simulink model "SdbRxSigProc"  (R2022b)
settings: Solver=FixedStepDiscrete  StartTime=0.0  StopTime=10.0  FixedStep=0.05  RelTol=1e-3
(part 1 of 4)
settings Model: Creator=blue.ge  LastModifiedBy=blue.ge
settings DataIOCC: SaveFormat=StructureWithTime
settings OptimizationCC: DefaultParameterBehavior=Inlined  EnhancedBackFolding=on  PassReuseOutputArgsAs=Individual arguments  ZeroExternalMemoryAtStartup=off  ZeroInternalMemoryAtStartup=off  OptimizeBlockOrder=speed  DifferentSizesBufferReuse=on  OptimizationCustomize=off  EfficientTunableParamExpr=on
settings DebuggingCC: ParameterTunabilityLossMsg=error
settings HardwareCC: ProdLongLongMode=on
settings ModelReferenceCC: ModelReferenceNumInstancesAllowed=Single
settings SFSimCC: MATLABDynamicMemAlloc=off
settings RTWCC: Description=AUTOSAR  SystemTargetFile=autosar.tlc  GenCodeOnly=on  TemplateMakefile=ert_default_tmf
settings CodeAppCC: ForceParamTrailComments=on  IgnoreCustomStorageClasses=off  OperatorAnnotations=on  SimulinkDataObjDesc=on  SFDataObjDesc=on  InsertBlockDesc=on
settings STFCustomTargetCC: TargetLangStandard=C99 (ISO)  MultiwordLength=256  ParMdlRefBuildCompliant=on  CompOptLevelCompliant=on  IncludeMdlTerminateFcn=off  CombineOutputUpdateFcns=on  SuppressErrorStatus=on  ERTCustomFileBanners=on  SupportNonFinite=off  SupportComplex=off  SupportContinuousTime=off  SupportNonInlinedSFcns=off  RemoveResetFunc=on  MATLABClassNameForMDSCustomization=Simulink.SoftwareTarget.ERTCustomization  AutosarCompliant=on  UseToolchainInfoCompliant=on  LookupTableObjectStructAxisOrder=2,1,3,4,...  LUTObjectStructOrderEvenSpacing=Size,Table,Breakpoints  SystemTargetFile=autosar.tlc
settings ERTTargetCC: TargetLangStandard=C99 (ISO)  ConcurrentExecutionCompliant=off  IncludeMdlTerminateFcn=off  SuppressErrorStatus=on  SupportNonFinite=off  SupportComplex=off  ModelStepFunctionPrototypeControlCompliant=off  CPPClassGenCompliant=off  AutosarCompliant=on  LookupTableObjectStructAxisOrder=2,1,3,4,...  LUTObjectStructOrderEvenSpacing=Size,Table,Breakpoints  InlinedParameterPlacement=NonHierarchical  ERTCustomFileTemplate=example_file_process.tlc

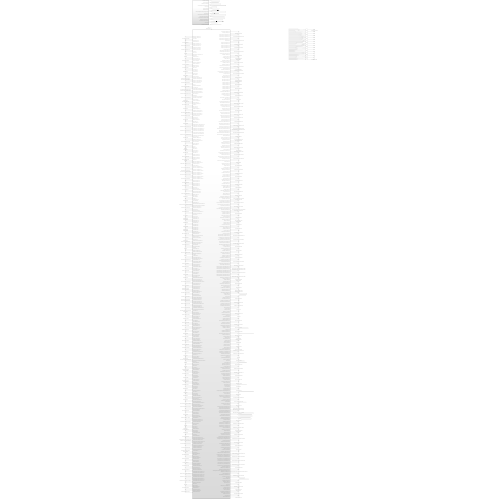
[thumbnail: Simulink's stored preview of the top-level diagram]
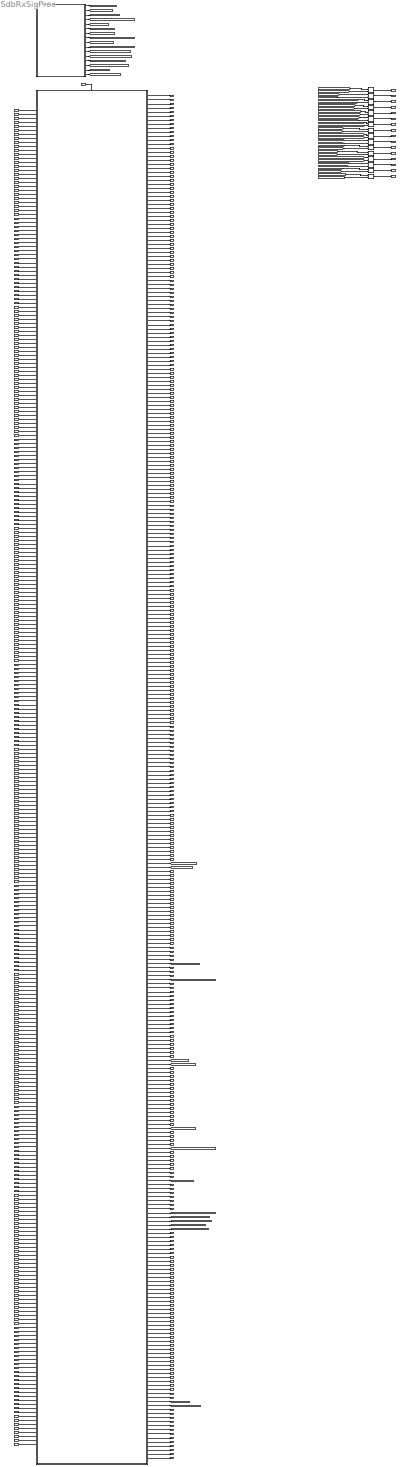
== system SdbRxSigProc ==
  SdbRxSigProc_Runnable : Inport  SampleTime=0.05  OutputFunctionCall=on
  ActvnOfWshrFrntSafe_ActvnOfWshrFrntSafe : Inport  Port=2  OutDataTypeStr=Enum: OnOffCrit1  PortDimensions=1
  AirTFromHeatgEstimd_AirTFromHeatgEstimd : Inport  Port=3  OutMin=-256  OutMax=255.9  OutDataTypeStr=T2  PortDimensions=1
  AmbTRaw_AmbTRaw : Inport  Port=4  OutDataTypeStr=Bus: AmbTWithQly  PortDimensions=1  BusOutputAsStruct=on
  BltLockStAtDrvr_BltLockStAtDrvr : Inport  Port=5  OutDataTypeStr=Bus: BltLockStFrnt  PortDimensions=1  BusOutputAsStruct=on
  BltLockStAtPass_BltLockStAtPass : Inport  Port=6  OutDataTypeStr=Bus: BltLockStFrnt  PortDimensions=1  BusOutputAsStruct=on
  BltLockStAtRowSecLe_BltLockStAtRowSecLe : Inport  Port=7  OutDataTypeStr=Bus: BltLockStRe  PortDimensions=1  BusOutputAsStruct=on
  BltLockStAtRowSecMid_BltLockStAtRowSecMid : Inport  Port=8  OutDataTypeStr=Bus: BltLockStRe  PortDimensions=1  BusOutputAsStruct=on
  BltLockStAtRowSecRi_BltLockStAtRowSecRi : Inport  Port=9  OutDataTypeStr=Bus: BltLockStRe  PortDimensions=1  BusOutputAsStruct=on
  BltLockStAtRowThrdLe_BltLockStAtRowThrdLe : Inport  Port=10  OutDataTypeStr=Bus: BltLockStRe  PortDimensions=1  BusOutputAsStruct=on
  BltLockStAtRowThrdRi_BltLockStAtRowThrdRi : Inport  Port=11  OutDataTypeStr=Bus: BltLockStRe  PortDimensions=1  BusOutputAsStruct=on
  BookTrvlClimaActvReq_BookTrvlClimaActvReq : Inport  Port=12  OutDataTypeStr=Enum: OnOffNoReq  PortDimensions=1
  CamSts_CamSts : Inport  Port=13  OutDataTypeStr=Bus: CamStsGrp  PortDimensions=1  BusOutputAsStruct=on
  CarTiGlb_CarTiGlb : Inport  Port=14  OutDataTypeStr=CarTiGlb  PortDimensions=1
  ClimaCfgForSnsrHumPrsnt1_ClimaCfgForSnsrHumPrsnt1 : Inport  Port=15  OutDataTypeStr=Boolean  PortDimensions=1
  ClimaCmd_ClimaCmd : Inport  Port=16  OutDataTypeStr=Enum: OkNotOk  PortDimensions=1
  ClimaPwrAllwd_ClimaPwrAllwd : Inport  Port=17  OutMin=0  OutMax=2047  OutDataTypeStr=Pwr30  PortDimensions=1
  CmpmtInAirPmAQI_CmpmtInAirPmAQI : Inport  Port=18  OutDataTypeStr=Enum: CmpmtAirPmLvl  PortDimensions=1
  CmpmtInAirPmDnsty_CmpmtInAirPmDnsty : Inport  Port=19  OutDataTypeStr=UInt10  PortDimensions=1
  CmptmtCoolgSts_CmptmtCoolgSts : Inport  Port=20  OutDataTypeStr=Enum: CmptmtCoolgSts  PortDimensions=1
  CmptmtFrntWindDewT_CmptmtFrntWindDewT : Inport  Port=21  OutDataTypeStr=TByCmptmtSnsrRelHum  PortDimensions=1
  CmptmtFrntWindT_CmptmtFrntWindT : Inport  Port=22  OutDataTypeStr=TByCmptmtSnsrRelHum  PortDimensions=1
  CmptmtRelHum_CmptmtRelHum : Inport  Port=23  OutMin=0  OutMax=100  OutDataTypeStr=Perc2  PortDimensions=1
  CoolgLoadGroup_CoolgLoadGroup : Inport  Port=24  OutDataTypeStr=Bus: CoolgLoad1  PortDimensions=1  BusOutputAsStruct=on
  DefrstSts_DefrstSts : Inport  Port=25  OutDataTypeStr=Bus: DefrstSts  PortDimensions=1  BusOutputAsStruct=on
  DoorDrvrSts_DoorDrvrSts : Inport  Port=26  OutDataTypeStr=Enum: DoorSts2  PortDimensions=1
  DoorLeReSts_DoorLeReSts : Inport  Port=27  OutDataTypeStr=Enum: DoorSts2  PortDimensions=1
  DoorPassSts_DoorPassSts : Inport  Port=28  OutDataTypeStr=Enum: DoorSts2  PortDimensions=1
  DoorRiReSts_DoorRiReSts : Inport  Port=29  OutDataTypeStr=Enum: DoorSts2  PortDimensions=1
  DrvModReq_DrvModReq : Inport  Port=30  OutDataTypeStr=Enum: DrvModReqType1  PortDimensions=1
  DrvrAirCdnrSetg_DrvrAirCdnrSetg : Inport  Port=31  OutDataTypeStr=Bus: DrvrAirCdnrSetg  PortDimensions=1  BusOutputAsStruct=on
  DrvrSeatHeatgActlPwrCns_DrvrSeatHeatgActlPwrCns : Inport  Port=32  OutDataTypeStr=UInt8  PortDimensions=1
  DrvrSeatHeatgDesPwrCns_DrvrSeatHeatgDesPwrCns : Inport  Port=33  OutDataTypeStr=UInt8  PortDimensions=1
  DrvrSeatVentnActlPwrCns_DrvrSeatVentnActlPwrCns : Inport  Port=34  OutDataTypeStr=UInt8  PortDimensions=1
  DrvrSeatVentnDesPwrCns_DrvrSeatVentnDesPwrCns : Inport  Port=35  OutDataTypeStr=UInt8  PortDimensions=1
  EgyCnsAllwdForClima_EgyCnsAllwdForClima : Inport  Port=36  OutDataTypeStr=Egy2  PortDimensions=1
  EngT_EngT : Inport  Port=37  OutDataTypeStr=Bus: EngT  PortDimensions=1  BusOutputAsStruct=on
  HMIClimaEgySaveReq_HMIClimaEgySaveReq : Inport  Port=38  OutDataTypeStr=Enum: VentnActr01BlckInd  PortDimensions=1
  HpcDeiceReq_HpcDeiceReq : Inport  Port=39  OutDataTypeStr=Enum: DeiceReq  PortDimensions=1
  HvacFanSts2HvacFanBattI2_HvacFanSts2HvacFanBattI2 : Inport  Port=40  OutMin=0  OutMax=63.25  OutDataTypeStr=BlowerReI  PortDimensions=1
  HvacFanSts2HvacFanMotU2_HvacFanSts2HvacFanMotU2 : Inport  Port=41  OutMin=0  OutMax=25.400000000000002  OutDataTypeStr=U8  PortDimensions=1
  HvacFanSts3HvacFanBattI_HvacFanSts3HvacFanBattI : Inport  Port=42  OutDataTypeStr=BlowerBattI  PortDimensions=1
  HvacFanSts3HvacFanSpdFd_HvacFanSts3HvacFanSpdFd : Inport  Port=43  OutDataTypeStr=BlowerSpd  PortDimensions=1
  HvPwrEgyPrio_HvPwrEgyPrio : Inport  Port=44  OutDataTypeStr=Enum: HvPwrEgyPrio  PortDimensions=1
  IHUAPSts_IHUAPSts : Inport  Port=45  OutDataTypeStr=Enum: OnOff1  PortDimensions=1
  IncomingAirQlyCtrlFromHmi_IncomingAirQlyCtrlFromHmi : Inport  Port=46  OutDataTypeStr=Enum: OnOff1  PortDimensions=1
  IncomingAirQlyPopUpReq_IncomingAirQlyPopUpReq : Inport  Port=47  OutDataTypeStr=Enum: OnOff1  PortDimensions=1
  InPm25FanErr_InPm25FanErr : Inport  Port=48  OutDataTypeStr=Enum: FanErr  PortDimensions=1
  InPm25SnsrElecErr_InPm25SnsrElecErr : Inport  Port=49  OutDataTypeStr=Enum: PmSnsrErrSts  PortDimensions=1
  InPm25SnsrSts_InPm25SnsrSts : Inport  Port=50  OutDataTypeStr=Enum: PmSnsrSts  PortDimensions=1
  InteCleanUnpleSmell_InteCleanUnpleSmell : Inport  Port=51  OutDataTypeStr=Enum: OnOff1  PortDimensions=1
  IsgMotT_IsgMotT : Inport  Port=52  OutDataTypeStr=T3  PortDimensions=1
  LeSolarData_LeSolarData : Inport  Port=53  OutDataTypeStr=Sunload2D  PortDimensions=1
  LockgCenSts_LockgCenSts : Inport  Port=54  OutDataTypeStr=Bus: LockgCenSts3  PortDimensions=1  BusOutputAsStruct=on
  OnBdChrgrHndlSts1_OnBdChrgrHndlSts1 : Inport  Port=55  OutDataTypeStr=Enum: OnBdChrgrHndlSts1  PortDimensions=1
  PassSeatHeatgActlPwrCns_PassSeatHeatgActlPwrCns : Inport  Port=56  OutDataTypeStr=UInt8  PortDimensions=1
  PassSeatHeatgDesPwrCns_PassSeatHeatgDesPwrCns : Inport  Port=57  OutDataTypeStr=UInt8  PortDimensions=1
  PassSeatVentnActlPwrCns_PassSeatVentnActlPwrCns : Inport  Port=58  OutDataTypeStr=UInt8  PortDimensions=1
  PassSeatVentnDesPwrCns_PassSeatVentnDesPwrCns : Inport  Port=59  OutDataTypeStr=UInt8  PortDimensions=1
  PostDrvgClimaReq_PostDrvgClimaReq : Inport  Port=60  OutDataTypeStr=Enum: OnOff1  PortDimensions=1
  RainfallAmnt_RainfallAmnt : Inport  Port=61  OutDataTypeStr=Enum: AmntSnsr  PortDimensions=1
  RelHumSnsrQf_RelHumSnsrQf : Inport  Port=62  OutDataTypeStr=Enum: DataQf  PortDimensions=1
  RemStrtHvCtrlReq_RemStrtHvCtrlReq : Inport  Port=63  OutDataTypeStr=Bus: ErsCmdRec  PortDimensions=1  BusOutputAsStruct=on
  RiSolarData_RiSolarData : Inport  Port=64  OutDataTypeStr=Sunload2D  PortDimensions=1
  SceneModSeld_SceneModSeld : Inport  Port=65  OutDataTypeStr=Enum: PatSeld  PortDimensions=1
  SeatHeatDurgClimaEnadFromTelm_SeatHeatDurgClimaEnadFromTelm : Inport  Port=66  OutDataTypeStr=Enum: SeatHeatDurgClimaEnad  PortDimensions=1
  SecRowSeatHeatgActlPwrCnsLe_SecRowSeatHeatgActlPwrCnsLe : Inport  Port=67  OutDataTypeStr=UInt8  PortDimensions=1
  SecRowSeatHeatgActlPwrCnsRi_SecRowSeatHeatgActlPwrCnsRi : Inport  Port=68  OutDataTypeStr=UInt8  PortDimensions=1
  SecRowSeatHeatgDesPwrCnsLe_SecRowSeatHeatgDesPwrCnsLe : Inport  Port=69  OutDataTypeStr=UInt8  PortDimensions=1
  SecRowSeatHeatgDesPwrCnsRi_SecRowSeatHeatgDesPwrCnsRi : Inport  Port=70  OutDataTypeStr=UInt8  PortDimensions=1
  SecRowSeatVentnActlPwrCnsLe_SecRowSeatVentnActlPwrCnsLe : Inport  Port=71  OutDataTypeStr=UInt8  PortDimensions=1
  SecRowSeatVentnActlPwrCnsRi_SecRowSeatVentnActlPwrCnsRi : Inport  Port=72  OutDataTypeStr=UInt8  PortDimensions=1
  SecRowSeatVentnDesPwrCnsLe_SecRowSeatVentnDesPwrCnsLe : Inport  Port=73  OutDataTypeStr=UInt8  PortDimensions=1
  SecRowSeatVentnDesPwrCnsRi_SecRowSeatVentnDesPwrCnsRi : Inport  Port=74  OutDataTypeStr=UInt8  PortDimensions=1
  SolarSnsrVluQf_SolarSnsrVluQf : Inport  Port=75  OutDataTypeStr=Enum: DataQf  PortDimensions=1
  SteerWhlHeatgDesPwr_SteerWhlHeatgDesPwr : Inport  Port=76  OutDataTypeStr=UInt8  PortDimensions=1
  SteerWhlHeatgPwrAct_SteerWhlHeatgPwrAct : Inport  Port=77  OutDataTypeStr=UInt8  PortDimensions=1
  StrtRemSts1WdStrtRemSts_StrtRemSts1WdStrtRemSts : Inport  Port=78  OutDataTypeStr=Enum: StrtRemSts1  PortDimensions=1
  SunCurtPosnSts_SunCurtPosnSts : Inport  Port=79  OutDataTypeStr=Enum: WinAndRoofAndCurtPosnTyp  PortDimensions=1
  SunRoofPosnSts_SunRoofPosnSts : Inport  Port=80  OutDataTypeStr=Enum: WinAndRoofAndCurtPosnTyp  PortDimensions=1
  TelmPM25Req_TelmPM25Req : Inport  Port=81  OutDataTypeStr=Enum: ReqSts1  PortDimensions=1
  TrSts_TrSts : Inport  Port=82  OutDataTypeStr=Enum: DoorSts2  PortDimensions=1
  VehBattU_VehBattU : Inport  Port=83  OutDataTypeStr=Bus: SysU  PortDimensions=1  BusOutputAsStruct=on
  VehSpdLgt_VehSpdLgt : Inport  Port=84  OutDataTypeStr=Bus: VehSpdLgtSafe1  PortDimensions=1  BusOutputAsStruct=on
  VehTiAndData_VehTiAndData : Inport  Port=85  OutDataTypeStr=Bus: DateTi30  PortDimensions=1  BusOutputAsStruct=on
  WadeProtnSts_WadeProtnSts : Inport  Port=86  OutDataTypeStr=Enum: OnOff1  PortDimensions=1
  WinGlbCmd_WinGlbCmd : Inport  Port=87  OutDataTypeStr=Enum: OpenClsGlbCmd  PortDimensions=1
  WinPosnStsAtDrvr_WinPosnStsAtDrvr : Inport  Port=88  OutDataTypeStr=Enum: WinAndRoofAndCurtPosnTyp  PortDimensions=1
  WinPosnStsAtPass_WinPosnStsAtPass : Inport  Port=89  OutDataTypeStr=Enum: WinAndRoofAndCurtPosnTyp  PortDimensions=1
  WinPosnStsAtReLe_WinPosnStsAtReLe : Inport  Port=90  OutDataTypeStr=Enum: WinAndRoofAndCurtPosnTyp  PortDimensions=1
  WinPosnStsAtReRi_WinPosnStsAtReRi : Inport  Port=91  OutDataTypeStr=Enum: WinAndRoofAndCurtPosnTyp  PortDimensions=1
  WipgInfo_WipgInfo : Inport  Port=92  OutDataTypeStr=Bus: WipgInfo  PortDimensions=1  BusOutputAsStruct=on
  VehModMngtGlbSafe1_VehModMngtGlbSafe1 : Inport  Port=93  OutDataTypeStr=Bus: VehModMngtGlbSafe1  PortDimensions=1  BusOutputAsStruct=on
  VehModMngtGlbSafe1_VehModMngtGlbSafe1_IsUpdated : Inport  Port=94  OutDataTypeStr=boolean  PortDimensions=1
  AACAirClngErrSts_AACAirClngErrSts : Inport  Port=95  OutDataTypeStr=Enum: AACAirClngErrSts  PortDimensions=1
  AACAirClngSts_AACAirClngSts : Inport  Port=96  OutDataTypeStr=Enum: AACAirClngSts  PortDimensions=1
  AFUFragraCh1AvlTiPrec_AFUFragraCh1AvlTiPrec : Inport  Port=97  OutMin=0  OutMax=100  OutDataTypeStr=Perc9  PortDimensions=1
  AFUFragraCh1AvlTiPrec_AFUFragraCh1AvlTiPrec_IsUpdated : Inport  Port=98  OutDataTypeStr=boolean  PortDimensions=1
  AFUFragraCh2AvlTiPrec_AFUFragraCh2AvlTiPrec : Inport  Port=99  OutMin=0  OutMax=100  OutDataTypeStr=Perc9  PortDimensions=1
  AFUFragraCh2AvlTiPrec_AFUFragraCh2AvlTiPrec_IsUpdated : Inport  Port=100  OutDataTypeStr=boolean  PortDimensions=1
  AFUFragraCh3AvlTiPrec_AFUFragraCh3AvlTiPrec : Inport  Port=101  OutMin=0  OutMax=100  OutDataTypeStr=Perc9  PortDimensions=1
  AFUFragraCh3AvlTiPrec_AFUFragraCh3AvlTiPrec_IsUpdated : Inport  Port=102  OutDataTypeStr=boolean  PortDimensions=1
  AFUFragraCh4AvlTiPrec_AFUFragraCh4AvlTiPrec : Inport  Port=103  OutMin=0  OutMax=100  OutDataTypeStr=Perc9  PortDimensions=1
  AFUFragraCh4AvlTiPrec_AFUFragraCh4AvlTiPrec_IsUpdated : Inport  Port=104  OutDataTypeStr=boolean  PortDimensions=1
  AFUFragraCh5AvlTiPrec_AFUFragraCh5AvlTiPrec : Inport  Port=105  OutMin=0  OutMax=100  OutDataTypeStr=Perc9  PortDimensions=1
  AFUFragraCh5AvlTiPrec_AFUFragraCh5AvlTiPrec_IsUpdated : Inport  Port=106  OutDataTypeStr=boolean  PortDimensions=1
  AirFragCh1OvrdDy_AirFragCh1OvrdDy : Inport  Port=107  OutDataTypeStr=Bus: DateTi30  PortDimensions=1  BusOutputAsStruct=on
  AirFragCh2OvrdDy_AirFragCh2OvrdDy : Inport  Port=108  OutDataTypeStr=Bus: DateTi30  PortDimensions=1  BusOutputAsStruct=on
  AirFragCh3OvrdDy_AirFragCh3OvrdDy : Inport  Port=109  OutDataTypeStr=Bus: DateTi30  PortDimensions=1  BusOutputAsStruct=on
  AirFragCh4OvrdDy_AirFragCh4OvrdDy : Inport  Port=110  OutDataTypeStr=Bus: DateTi30  PortDimensions=1  BusOutputAsStruct=on
  AirFragCh5OvrdDy_AirFragCh5OvrdDy : Inport  Port=111  OutDataTypeStr=Bus: DateTi30  PortDimensions=1  BusOutputAsStruct=on
  AirPtcEnad_AirPtcEnad : Inport  Port=112  OutDataTypeStr=Boolean  PortDimensions=1
  AirPtcHVPwrActl_AirPtcHVPwrActl : Inport  Port=113  OutDataTypeStr=Pwr5  PortDimensions=1
  AirPtcHVPwrActlFristLe_AirPtcHVPwrActlFristLe : Inport  Port=114  OutMin=0  OutMax=20460  OutDataTypeStr=Pwr3  PortDimensions=1
  AirPtcHVPwrActlFristRi_AirPtcHVPwrActlFristRi : Inport  Port=115  OutMin=0  OutMax=20460  OutDataTypeStr=Pwr3  PortDimensions=1
  AirPtcHVPwrActlSecLe_AirPtcHVPwrActlSecLe : Inport  Port=116  OutMin=0  OutMax=20460  OutDataTypeStr=Pwr3  PortDimensions=1
  AirPtcModeReq_AirPtcModeReq : Inport  Port=117  OutDataTypeStr=Enum: AirPtcModeReq  PortDimensions=1
  AirSrcStsFb_AirSrcStsFb : Inport  Port=118  OutMin=0  OutMax=3  OutDataTypeStr=UInt2  PortDimensions=1
  AltitudeInfrom_AltitudeInfrom : Inport  Port=119  OutDataTypeStr=UInt15  PortDimensions=1
  AMBSts_AMBSts : Inport  Port=120  OutDataTypeStr=Bus: AMBStsgrp  PortDimensions=1  BusOutputAsStruct=on
  BackflushVlvErr_BackflushVlvErr : Inport  Port=121  OutMin=0  OutMax=3  OutDataTypeStr=UInt2  PortDimensions=1
  BusCurrentFb_BusCurrentFb : Inport  Port=122  OutDataTypeStr=PackCurrent  PortDimensions=1
  ChgFilElmWarn_ChgFilElmWarn : Inport  Port=123  OutMin=0  OutMax=3  OutDataTypeStr=UInt2  PortDimensions=1
  ClimaCfgForDrvrSeatVentWthSmf2_ClimaCfgForDrvrSeatVentWthSmf2 : Inport  Port=124  OutDataTypeStr=UInt8  PortDimensions=1
  ClimaCfgForPassSeatVentWthSmf2_ClimaCfgForPassSeatVentWthSmf2 : Inport  Port=125  OutDataTypeStr=UInt8  PortDimensions=1
  CmptmtOxygenConcQF_CmptmtOxygenConcQF : Inport  Port=126  OutDataTypeStr=Enum: Qf1  PortDimensions=1
  CmptmtOxygenConcVlu_CmptmtOxygenConcVlu : Inport  Port=127  OutMin=0  OutMax=100  OutDataTypeStr=Perc9  PortDimensions=1
  CoolgFanErr_CoolgFanErr : Inport  Port=128  OutMin=0  OutMax=3  OutDataTypeStr=UInt2  PortDimensions=1
  CourseDirFromNav_CourseDirFromNav : Inport  Port=129  OutDataTypeStr=Bus: NavDir  PortDimensions=1  BusOutputAsStruct=on
  CurrentCongestionInformation_CurrentCongestionInformation : Inport  Port=130  OutMin=0  OutMax=15  OutDataTypeStr=UInt4  PortDimensions=1
  CurrentTunnelInformation_CurrentTunnelInformation : Inport  Port=131  OutDataTypeStr=Enum: OnOffNoReq  PortDimensions=1
  DcDcActvd_DcDcActvd : Inport  Port=132  OutDataTypeStr=Enum: DcDcActvd  PortDimensions=1
  DcDcPwrLim_DcDcPwrLim : Inport  Port=133  OutDataTypeStr=Boolean  PortDimensions=1
  DHUResdSig10_DHUResdSig10 : Inport  Port=134  OutDataTypeStr=UInt32  PortDimensions=1
  DHUResdSig11_DHUResdSig11 : Inport  Port=135  OutDataTypeStr=Unit64  PortDimensions=1
  DHUResdSig12_DHUResdSig12 : Inport  Port=136  OutDataTypeStr=Unit64  PortDimensions=1
  DHUResdSig13_DHUResdSig13 : Inport  Port=137  OutDataTypeStr=UInt32  PortDimensions=1
  DHUResdSig3_DHUResdSig3 : Inport  Port=138  OutDataTypeStr=UInt16  PortDimensions=1
  DHUResdSig4_DHUResdSig4 : Inport  Port=139  OutDataTypeStr=UInt16  PortDimensions=1
  DHUResdSig5_DHUResdSig5 : Inport  Port=140  OutDataTypeStr=UInt32  PortDimensions=1
  DHUResdSig6_DHUResdSig6 : Inport  Port=141  OutDataTypeStr=Unit64  PortDimensions=1
  DHUResdSig7_DHUResdSig7 : Inport  Port=142  OutDataTypeStr=Unit64  PortDimensions=1
  DHUResdSig8_DHUResdSig8 : Inport  Port=143  OutDataTypeStr=UInt16  PortDimensions=1
  DHUResdSig9_DHUResdSig9 : Inport  Port=144  OutDataTypeStr=UInt16  PortDimensions=1
  DispHvBattLvlOfChrg_DispHvBattLvlOfChrg : Inport  Port=145  OutMin=0  OutMax=102.30000000000001  OutDataTypeStr=EcoDrvInfo  PortDimensions=1
  DropTopPosnSts_DropTopPosnSts : Inport  Port=146  OutDataTypeStr=Enum: PosnSts  PortDimensions=1
  DrvModReqType1ForCompile_DrvModReqType1ForCompile : Inport  Port=147  OutDataTypeStr=Enum: DrvModReqType1  PortDimensions=1
  DrvrSeatPosPerc_DrvrSeatPosPerc : Inport  Port=148  OutDataTypeStr=Bus: SeatPosPercQF  PortDimensions=1  BusOutputAsStruct=on
  DrvrSeatPosPerc_DrvrSeatPosPerc_IsUpdated : Inport  Port=149  OutDataTypeStr=boolean  PortDimensions=1
  DrvrSeatSts_DrvrSeatSts : Inport  Port=150  OutDataTypeStr=Enum: OccptPresSt1  PortDimensions=1
  DrvrSeatVentnActlPwrCns2_DrvrSeatVentnActlPwrCns2 : Inport  Port=151  OutDataTypeStr=UInt8  PortDimensions=1
  DrvrSeatVentnDesPwrCns2_DrvrSeatVentnDesPwrCns2 : Inport  Port=152  OutDataTypeStr=UInt8  PortDimensions=1
  DrvrSeatVentnLvlSts2_DrvrSeatVentnLvlSts2 : Inport  Port=153  OutDataTypeStr=Enum: SeatClimaLvl  PortDimensions=1
  EngSt1WdSts_EngSt1WdSts : Inport  Port=154  OutDataTypeStr=Bus: EngSt1WdSts  PortDimensions=1  BusOutputAsStruct=on
  EngSt1WdSts_EngSt1WdSts_IsUpdated : Inport  Port=155  OutDataTypeStr=boolean  PortDimensions=1
  FctvSts_FctvSts : Inport  Port=156  OutDataTypeStr=Bus: ValveSts  PortDimensions=1  BusOutputAsStruct=on
  FloatDrvingActv_FloatDrvingActv : Inport  Port=157  OutDataTypeStr=Bus: FloatDrvingActv  PortDimensions=1  BusOutputAsStruct=on
  FrntAirPtcHVPwrActl_FrntAirPtcHVPwrActl : Inport  Port=158  OutMin=0  OutMax=20460  OutDataTypeStr=Pwr3  PortDimensions=1
  FrntAirPtcHVPwrActl_FrntAirPtcHVPwrActl_IsUpdated : Inport  Port=159  OutDataTypeStr=boolean  PortDimensions=1
  HighTempCleanHmiReq_HighTempCleanHmiReq : Inport  Port=160  OutDataTypeStr=Enum: NoReqOnOff  PortDimensions=1
  HmiAC_HmiAC : Inport  Port=161  OutDataTypeStr=Enum: HmiCmptmtCoolgReq  PortDimensions=1
  HmiAC_HmiAC_IsUpdated : Inport  Port=162  OutDataTypeStr=boolean  PortDimensions=1
  HMIAirCdnrAutDrySet_HMIAirCdnrAutDrySet : Inport  Port=163  OutDataTypeStr=Enum: OnOff1  PortDimensions=1
  HMIAirCdnrAutDrySet_HMIAirCdnrAutDrySet_IsUpdated : Inport  Port=164  OutDataTypeStr=boolean  PortDimensions=1
  HmiAirDistbnRow1_HmiAirDistbnRow1 : Inport  Port=165  OutDataTypeStr=Enum: HmiCmptmtAirDistbnFrnt  PortDimensions=1
  HmiAirDistbnRow1_HmiAirDistbnRow1_IsUpdated : Inport  Port=166  OutDataTypeStr=boolean  PortDimensions=1
  HmiAirDistbnRow1Le_HmiAirDistbnRow1Le : Inport  Port=167  OutDataTypeStr=Enum: HmiCmptmtAirDistbnFrnt  PortDimensions=1
  HmiAirDistbnRow1Ri_HmiAirDistbnRow1Ri : Inport  Port=168  OutDataTypeStr=Enum: HmiCmptmtAirDistbnFrnt  PortDimensions=1
  HmiAirDistbnRow2_HmiAirDistbnRow2 : Inport  Port=169  OutDataTypeStr=Enum: HmiCmptmtAirDistbnFrnt  PortDimensions=1
  HmiAirDistbnRow2_HmiAirDistbnRow2_IsUpdated : Inport  Port=170  OutDataTypeStr=boolean  PortDimensions=1
  HmiAutoRow1_HmiAutoRow1 : Inport  Port=171  OutDataTypeStr=Enum: OnOff1  PortDimensions=1
  HmiAutoRow1_HmiAutoRow1_IsUpdated : Inport  Port=172  OutDataTypeStr=boolean  PortDimensions=1
  HmiAutoRow1Le_HmiAutoRow1Le : Inport  Port=173  OutDataTypeStr=Enum: OnOff1  PortDimensions=1
  HmiAutoRow1Ri_HmiAutoRow1Ri : Inport  Port=174  OutDataTypeStr=Enum: OnOff1  PortDimensions=1
  HmiAutoRow2_HmiAutoRow2 : Inport  Port=175  OutDataTypeStr=Enum: OnOff1  PortDimensions=1
  HmiAutoRow2_HmiAutoRow2_IsUpdated : Inport  Port=176  OutDataTypeStr=boolean  PortDimensions=1
  HmiAutOxySet_HmiAutOxySet : Inport  Port=177  OutDataTypeStr=Enum: OnOff1  PortDimensions=1
  HMIBluetoothCallToAdjAirVol_HMIBluetoothCallToAdjAirVol : Inport  Port=178  OutDataTypeStr=Enum: OnOffNoReq  PortDimensions=1
  HmiCarLockACRecircSet_HmiCarLockACRecircSet : Inport  Port=179  OutMin=0  OutMax=3  OutDataTypeStr=UInt2  PortDimensions=1
  HmiCbnOverHeatPrevnReq_HmiCbnOverHeatPrevnReq : Inport  Port=180  OutDataTypeStr=Enum: OnOffNoReq  PortDimensions=1
  HmiCbnOverHeatPrevnReq_HmiCbnOverHeatPrevnReq_IsUpdated : Inport  Port=181  OutDataTypeStr=boolean  PortDimensions=1
  HmiClimaRow1LePwr_HmiClimaRow1LePwr : Inport  Port=182  OutDataTypeStr=Enum: OnOff1  PortDimensions=1
  HmiClimaRow1Pwr_HmiClimaRow1Pwr : Inport  Port=183  OutDataTypeStr=Enum: OnOff1  PortDimensions=1
  HmiClimaRow1RiPwr_HmiClimaRow1RiPwr : Inport  Port=184  OutDataTypeStr=Enum: OnOff1  PortDimensions=1
  HmiClimaRow2Pwr_HmiClimaRow2Pwr : Inport  Port=185  OutDataTypeStr=Enum: OnOff1  PortDimensions=1
  HmiClimaRow3Pwr_HmiClimaRow3Pwr : Inport  Port=186  OutDataTypeStr=Enum: OnOff1  PortDimensions=1
  HMIClimaVentSet_HMIClimaVentSet : Inport  Port=187  OutDataTypeStr=Enum: OnOff1  PortDimensions=1
  HmiCmptmtAirDistbnFrnt_HmiCmptmtAirDistbnFrnt : Inport  Port=188  OutDataTypeStr=Enum: HmiCmptmtAirDistbnFrnt  PortDimensions=1
  HmiDrvrHeadPosn_HmiDrvrHeadPosn : Inport  Port=189  OutDataTypeStr=Bus: GazeDirOri  PortDimensions=1  BusOutputAsStruct=on
  HmiEcoClimaReq_HmiEcoClimaReq : Inport  Port=190  OutDataTypeStr=Enum: OnOffNoReq  PortDimensions=1
  HmiEcoClimaReq_HmiEcoClimaReq_IsUpdated : Inport  Port=191  OutDataTypeStr=boolean  PortDimensions=1
  HmiElecAirBodyVentLebtn_HmiElecAirBodyVentLebtn : Inport  Port=192  OutMin=0  OutMax=15  OutDataTypeStr=UInt4  PortDimensions=1
  HmiElecAirBodyVentRibtn_HmiElecAirBodyVentRibtn : Inport  Port=193  OutMin=0  OutMax=15  OutDataTypeStr=UInt4  PortDimensions=1
  HmiElecAirConsolePosn_HmiElecAirConsolePosn : Inport  Port=194  OutDataTypeStr=Bus: PosIndct  PortDimensions=1  BusOutputAsStruct=on
  HmiElecAirConsoleSwtLe_HmiElecAirConsoleSwtLe : Inport  Port=195  OutDataTypeStr=Enum: OnOff1  PortDimensions=1
  HmiElecAirConsoleSwtRi_HmiElecAirConsoleSwtRi : Inport  Port=196  OutDataTypeStr=Enum: OnOff1  PortDimensions=1
  HmiElecAirDirModeSecRowReq_HmiElecAirDirModeSecRowReq : Inport  Port=197  OutDataTypeStr=Bus: DirModReq1  PortDimensions=1  BusOutputAsStruct=on
  HmiElecAirDirSwngSecLe_HmiElecAirDirSwngSecLe : Inport  Port=198  OutDataTypeStr=Enum: OnOff1  PortDimensions=1
  HmiElecAirDirSwngSecRi_HmiElecAirDirSwngSecRi : Inport  Port=199  OutDataTypeStr=Enum: OnOff1  PortDimensions=1
  HmiElecAirDrvrNatureWindReq_HmiElecAirDrvrNatureWindReq : Inport  Port=200  OutDataTypeStr=Enum: OnOff1  PortDimensions=1
  HmiElecAirPassNatureWindReq_HmiElecAirPassNatureWindReq : Inport  Port=201  OutDataTypeStr=Enum: OnOff1  PortDimensions=1
  HMIFACSwt_HMIFACSwt : Inport  Port=202  OutDataTypeStr=Enum: OnOff1  PortDimensions=1
  HmiFanLvlRow1_HmiFanLvlRow1 : Inport  Port=203  OutDataTypeStr=Enum: HmiHvacFanLvl  PortDimensions=1
  HmiFanLvlRow1_HmiFanLvlRow1_IsUpdated : Inport  Port=204  OutDataTypeStr=boolean  PortDimensions=1
  HmiFanLvlRow1Le_HmiFanLvlRow1Le : Inport  Port=205  OutDataTypeStr=Enum: HmiHvacFanLvl  PortDimensions=1
  HmiFanLvlRow1Ri_HmiFanLvlRow1Ri : Inport  Port=206  OutDataTypeStr=Enum: HmiHvacFanLvl  PortDimensions=1
  HmiFanLvlRow2_HmiFanLvlRow2 : Inport  Port=207  OutDataTypeStr=Enum: HmiHvacFanLvl  PortDimensions=1
  HmiFanLvlRow2_HmiFanLvlRow2_IsUpdated : Inport  Port=208  OutDataTypeStr=boolean  PortDimensions=1
  HmiFragraCh_HmiFragraCh : Inport  Port=209  OutDataTypeStr=Enum: ChSet  PortDimensions=1
  HmiFragraCh_HmiFragraCh_IsUpdated : Inport  Port=210  OutDataTypeStr=boolean  PortDimensions=1
  HmiFragraLvl_HmiFragraLvl : Inport  Port=211  OutDataTypeStr=Enum: HmiFragraLvlReq  PortDimensions=1
  HmiFragraLvl_HmiFragraLvl_IsUpdated : Inport  Port=212  OutDataTypeStr=boolean  PortDimensions=1
  HMIIonizerAirCdnr_HMIIonizerAirCdnr : Inport  Port=213  OutDataTypeStr=Enum: OnOff1  PortDimensions=1
  HMIIonizerAirCdnr_HMIIonizerAirCdnr_IsUpdated : Inport  Port=214  OutDataTypeStr=boolean  PortDimensions=1
  HMILBRACStrtSet_HMILBRACStrtSet : Inport  Port=215  OutDataTypeStr=Enum: OnOff1  PortDimensions=1
  HmiMaxAC_HmiMaxAC : Inport  Port=216  OutDataTypeStr=Enum: OnOff1  PortDimensions=1
  HmiMaxAC_HmiMaxAC_IsUpdated : Inport  Port=217  OutDataTypeStr=boolean  PortDimensions=1
  HmiMaxDefrst_HmiMaxDefrst : Inport  Port=218  OutDataTypeStr=Enum: OnOff1  PortDimensions=1
  HmiMaxDefrst_HmiMaxDefrst_IsUpdated : Inport  Port=219  OutDataTypeStr=boolean  PortDimensions=1
  HmiOxyinCarSwt_HmiOxyinCarSwt : Inport  Port=220  OutDataTypeStr=Enum: NoReqOnOff  PortDimensions=1
  HmiParkVentSet_HmiParkVentSet : Inport  Port=221  OutDataTypeStr=Enum: OnOff1  PortDimensions=1
  HmiParkVentTmrSet_HmiParkVentTmrSet : Inport  Port=222  OutMin=0  OutMax=31  OutDataTypeStr=TimeHr5  PortDimensions=1
  HmiPassHeadPosn_HmiPassHeadPosn : Inport  Port=223  OutDataTypeStr=Bus: GazeDirOri  PortDimensions=1  BusOutputAsStruct=on
  HmiPM25PopUpResp_HmiPM25PopUpResp : Inport  Port=224  OutDataTypeStr=Enum: HmiPM25PopUpResp  PortDimensions=1
  HmiPM25PopUpResp_HmiPM25PopUpResp_IsUpdated : Inport  Port=225  OutDataTypeStr=boolean  PortDimensions=1
  HmiPM25PopUpSet_HmiPM25PopUpSet : Inport  Port=226  OutDataTypeStr=Enum: OnOff1  PortDimensions=1
  HmiPM25PopUpSet_HmiPM25PopUpSet_IsUpdated : Inport  Port=227  OutDataTypeStr=boolean  PortDimensions=1
  HmiSmrtWindFolwSet_HmiSmrtWindFolwSet : Inport  Port=228  OutDataTypeStr=Enum: OnOff1  PortDimensions=1
  HMIThermoTankDoorOpen_HMIThermoTankDoorOpen : Inport  Port=229  OutDataTypeStr=Enum: OnOffNoReq  PortDimensions=1
  HMIThermoTankEcoSw_HMIThermoTankEcoSw : Inport  Port=230  OutDataTypeStr=Enum: NoReqOnOff  PortDimensions=1
  HMIThermoTankT_HMIThermoTankT : Inport  Port=231  OutDataTypeStr=TempAtTime  PortDimensions=1
  HmiThermoTSteDeoNewSwt_HmiThermoTSteDeoNewSwt : Inport  Port=232  OutDataTypeStr=Enum: OnOffNoReq  PortDimensions=1
  HmiThermoTSteDeoSwt_HmiThermoTSteDeoSwt : Inport  Port=233  OutDataTypeStr=Enum: OnOff1  PortDimensions=1
  HmiTSyncn_HmiTSyncn : Inport  Port=234  OutDataTypeStr=Enum: OnOff1  PortDimensions=1
  HmiTSyncn_HmiTSyncn_IsUpdated : Inport  Port=235  OutDataTypeStr=boolean  PortDimensions=1
  HmiUVSterilSet_HmiUVSterilSet : Inport  Port=236  OutDataTypeStr=Enum: OnOff1  PortDimensions=1
  HmiWinterLockCarPrevnIceSet_HmiWinterLockCarPrevnIceSet : Inport  Port=237  OutDataTypeStr=Enum: OnOff1  PortDimensions=1
  HmiWinterLockCarPrevnIceSet_HmiWinterLockCarPrevnIceSet_IsUpdated : Inport  Port=238  OutDataTypeStr=boolean  PortDimensions=1
  HoodSts_HoodSts : Inport  Port=239  OutDataTypeStr=Enum: DoorSts2  PortDimensions=1
  HvBattLimnIndcn_HvBattLimnIndcn : Inport  Port=240  OutDataTypeStr=UInt16  PortDimensions=1
  InflateAirSrcSwt_InflateAirSrcSwt : Inport  Port=241  OutDataTypeStr=Enum: OnOffNoReq  PortDimensions=1
  InpVoltFb_InpVoltFb : Inport  Port=242  OutMin=0  OutMax=1638.3000000000002  OutDataTypeStr=RlyMeaPointVolt  PortDimensions=1
  LiOprnMod_LiOprnMod : Inport  Port=243  OutDataTypeStr=Enum: LiOperMod  PortDimensions=1
  LonglifeBatteryReq_LonglifeBatteryReq : Inport  Port=244  OutDataTypeStr=Enum: BatteryReq  PortDimensions=1
  McuOvrTempErr_McuOvrTempErr : Inport  Port=245  OutMin=0  OutMax=3  OutDataTypeStr=UInt2  PortDimensions=1
  McuTempFb_McuTempFb : Inport  Port=246  OutMin=-256  OutMax=255.9  OutDataTypeStr=MvBattTemp  PortDimensions=1
  McuTempSnsrErr_McuTempSnsrErr : Inport  Port=247  OutMin=0  OutMax=3  OutDataTypeStr=UInt2  PortDimensions=1
  MoleculeVlv1_MoleculeVlv1 : Inport  Port=248  OutMin=0  OutMax=3  OutDataTypeStr=UInt2  PortDimensions=1
  MoleculeVlv2_MoleculeVlv2 : Inport  Port=249  OutMin=0  OutMax=3  OutDataTypeStr=UInt2  PortDimensions=1
  MosOvrTempErr_MosOvrTempErr : Inport  Port=250  OutMin=0  OutMax=3  OutDataTypeStr=UInt2  PortDimensions=1
  MosTempFb_MosTempFb : Inport  Port=251  OutMin=-256  OutMax=255.9  OutDataTypeStr=MvBattTemp  PortDimensions=1
  MosTempSnsrErr_MosTempSnsrErr : Inport  Port=252  OutMin=0  OutMax=3  OutDataTypeStr=UInt2  PortDimensions=1
  MotStsFrmOGM_MotStsFrmOGM : Inport  Port=253  OutDataTypeStr=UInt8  PortDimensions=1
  MotTempFb_MotTempFb : Inport  Port=254  OutMin=-256  OutMax=255.9  OutDataTypeStr=MvBattTemp  PortDimensions=1
  MotTempSnsrErr_MotTempSnsrErr : Inport  Port=255  OutMin=0  OutMax=3  OutDataTypeStr=UInt2  PortDimensions=1
  OGMCmprSpdFb_OGMCmprSpdFb : Inport  Port=256  OutDataTypeStr=UInt16  PortDimensions=1
  OGMFlowFb_OGMFlowFb : Inport  Port=257  OutMin=0  OutMax=51.1  OutDataTypeStr=CooltFlow2  PortDimensions=1
  OGMHwVers_OGMHwVers : Inport  Port=258  OutDataTypeStr=UInt16  PortDimensions=1
  OGMIntErr_OGMIntErr : Inport  Port=259  OutDataTypeStr=Enum: Err1  PortDimensions=1
  OGMOvrVoltRangErr_OGMOvrVoltRangErr : Inport  Port=260  OutDataTypeStr=Enum: Err1  PortDimensions=1
  OGMRunTi_OGMRunTi : Inport  Port=261  OutDataTypeStr=UInt32  PortDimensions=1
  OGMSwVers_OGMSwVers : Inport  Port=262  OutDataTypeStr=UInt16  PortDimensions=1
  OGMTempErr_OGMTempErr : Inport  Port=263  OutDataTypeStr=Enum: TempErr  PortDimensions=1
  OGMUndVoltRangErr_OGMUndVoltRangErr : Inport  Port=264  OutDataTypeStr=Enum: Err1  PortDimensions=1
  OxygenConcFbFrmOGM_OxygenConcFbFrmOGM : Inport  Port=265  OutMin=0  OutMax=100  OutDataTypeStr=Perc9  PortDimensions=1
  OxygenConcFlt_OxygenConcFlt : Inport  Port=266  OutMin=0  OutMax=3  OutDataTypeStr=UInt2  PortDimensions=1
  OxygenConcSnsrElecErr_OxygenConcSnsrElecErr : Inport  Port=267  OutDataTypeStr=Enum: OxygenConcSnsrElecErr  PortDimensions=1
  OxygenConcSnsrIntErr_OxygenConcSnsrIntErr : Inport  Port=268  OutDataTypeStr=Enum: Err1  PortDimensions=1
  OxygenConcSnsrOvrVoltRangErr_OxygenConcSnsrOvrVoltRangErr : Inport  Port=269  OutDataTypeStr=Enum: Err1  PortDimensions=1
  OxygenConcSnsrSts_OxygenConcSnsrSts : Inport  Port=270  OutDataTypeStr=Enum: PmSnsrSts  PortDimensions=1
  OxygenConcSnsrStsFrmOGM_OxygenConcSnsrStsFrmOGM : Inport  Port=271  OutMin=0  OutMax=3  OutDataTypeStr=UInt2  PortDimensions=1
  OxygenConcSnsrTempErr_OxygenConcSnsrTempErr : Inport  Port=272  OutDataTypeStr=Enum: TempErr  PortDimensions=1
  OxygenConcSnsrUndVoltRangErr_OxygenConcSnsrUndVoltRangErr : Inport  Port=273  OutDataTypeStr=Enum: Err1  PortDimensions=1
  OxygenGenrErrInfo_OxygenGenrErrInfo : Inport  Port=274  OutDataTypeStr=Enum: OxygengenrErrInfo  PortDimensions=1
  OxygenSplyAvlSts_OxygenSplyAvlSts : Inport  Port=275  OutDataTypeStr=Enum: OxygenSplyAvlSts  PortDimensions=1
  OxygenSplyErr_OxygenSplyErr : Inport  Port=276  OutDataTypeStr=Enum: Err1  PortDimensions=1
  OxygenTubeBlk_OxygenTubeBlk : Inport  Port=277  OutMin=0  OutMax=3  OutDataTypeStr=UInt2  PortDimensions=1
  PassSeatPosPerc_PassSeatPosPerc : Inport  Port=278  OutDataTypeStr=Bus: SeatPosPercQF  PortDimensions=1  BusOutputAsStruct=on
  PassSeatPosPerc_PassSeatPosPerc_IsUpdated : Inport  Port=279  OutDataTypeStr=boolean  PortDimensions=1
  PassSeatSts2_PassSeatSts2 : Inport  Port=280  OutDataTypeStr=Enum: OccptPresSt1  PortDimensions=1
  PassSeatVentnActlPwrCns2_PassSeatVentnActlPwrCns2 : Inport  Port=281  OutDataTypeStr=UInt8  PortDimensions=1
  PassSeatVentnDesPwrCns2_PassSeatVentnDesPwrCns2 : Inport  Port=282  OutDataTypeStr=UInt8  PortDimensions=1
  PassSeatVentnLvlSts2_PassSeatVentnLvlSts2 : Inport  Port=283  OutDataTypeStr=Enum: SeatClimaLvl  PortDimensions=1
  PhaseCurrentFb_PhaseCurrentFb : Inport  Port=284  OutDataTypeStr=PackCurrent  PortDimensions=1
  PosnBrief_PosnBrief : Inport  Port=285  OutDataTypeStr=Bus: Posn  PortDimensions=1  BusOutputAsStruct=on
  PressureFb_PressureFb : Inport  Port=286  OutMin=0  OutMax=400  OutDataTypeStr=Pressure2  PortDimensions=1
  PressureFlt_PressureFlt : Inport  Port=287  OutMin=0  OutMax=3  OutDataTypeStr=UInt2  PortDimensions=1
  PressureSnsrErr_PressureSnsrErr : Inport  Port=288  OutMin=0  OutMax=3  OutDataTypeStr=UInt2  PortDimensions=1
  RefrLpSwSts_RefrLpSwSts : Inport  Port=289  OutDataTypeStr=Enum: CloseOpen  PortDimensions=1
  RefrtorVlvEna_RefrtorVlvEna : Inport  Port=290  OutDataTypeStr=Enum: OnOff1  PortDimensions=1
  ResrvdSigForCCM2_ResrvdSigForCCM2 : Inport  Port=291  OutDataTypeStr=BattIdTbl  PortDimensions=1
  RoadIncln_RoadIncln : Inport  Port=292  OutDataTypeStr=Bus: RoadInclnRec1  PortDimensions=1  BusOutputAsStruct=on
  RuranACModeSet_RuranACModeSet : Inport  Port=293  OutDataTypeStr=Enum: RuranACModeSet  PortDimensions=1
  SeatBackAngleRowFirstDrvr_SeatBackAngleRowFirstDrvr : Inport  Port=294  OutMin=0  OutMax=180  OutDataTypeStr=AngleDeg180  PortDimensions=1
  SeatBackAngleRowFirstDrvr_SeatBackAngleRowFirstDrvr_IsUpdated : Inport  Port=295  OutDataTypeStr=boolean  PortDimensions=1
  SeatBackAngleRowFirstPass_SeatBackAngleRowFirstPass : Inport  Port=296  OutMin=0  OutMax=180  OutDataTypeStr=AngleDeg180  PortDimensions=1
  SeatBackAngleRowFirstPass_SeatBackAngleRowFirstPass_IsUpdated : Inport  Port=297  OutDataTypeStr=boolean  PortDimensions=1
  SeatOccptAtRowSecDrSide2_SeatOccptAtRowSecDrSide2 : Inport  Port=298  OutDataTypeStr=Enum: OccptPresSt1  PortDimensions=1
  SeatOccptAtRowSecMid2_SeatOccptAtRowSecMid2 : Inport  Port=299  OutDataTypeStr=Enum: OccptPresSt1  PortDimensions=1
  SeatOccptAtRowSecPasSide2_SeatOccptAtRowSecPasSide2 : Inport  Port=300  OutDataTypeStr=Enum: OccptPresSt1  PortDimensions=1
  SeatOccptAtRowThrdLe2_SeatOccptAtRowThrdLe2 : Inport  Port=301  OutDataTypeStr=Enum: OccptPresSt1  PortDimensions=1
  SeatOccptAtRowThrdRi2_SeatOccptAtRowThrdRi2 : Inport  Port=302  OutDataTypeStr=Enum: OccptPresSt1  PortDimensions=1
  SportModeThmgtRequest_SportModeThmgtRequest : Inport  Port=303  OutDataTypeStr=Enum: ThmgtLevel  PortDimensions=1
  TCSCooltFlow_TCSCooltFlow : Inport  Port=304  OutDataTypeStr=CooltFlow1  PortDimensions=1
  TCSCooltT_TCSCooltT : Inport  Port=305  OutDataTypeStr=TempAtTime  PortDimensions=1
  TcsDoorEleBailReq_TcsDoorEleBailReq : Inport  Port=306  OutDataTypeStr=Enum: WhlMotSysCluReq  PortDimensions=1
  TcsFanReq_TcsFanReq : Inport  Port=307  OutMin=0  OutMax=15  OutDataTypeStr=UInt4  PortDimensions=1
  TcsModSwtReq_TcsModSwtReq : Inport  Port=308  OutDataTypeStr=Enum: TelmThermoTankReq  PortDimensions=1
  TcsOvrheatdProtnSts_TcsOvrheatdProtnSts : Inport  Port=309  OutMin=0  OutMax=3  OutDataTypeStr=UInt2  PortDimensions=1
  TcsSemiconReq_TcsSemiconReq : Inport  Port=310  OutMin=0  OutMax=15  OutDataTypeStr=UInt4  PortDimensions=1
  TcsTmrOverReq_TcsTmrOverReq : Inport  Port=311  OutMin=0  OutMax=31  OutDataTypeStr=TimeHr5  PortDimensions=1
  TcsTNowCtrlReq_TcsTNowCtrlReq : Inport  Port=312  OutDataTypeStr=TempAtTime  PortDimensions=1
  TcsTopDoorEleBailReq_TcsTopDoorEleBailReq : Inport  Port=313  OutDataTypeStr=Enum: WhlMotSysCluReq  PortDimensions=1
  TelmOxygenValReq_TelmOxygenValReq : Inport  Port=314  OutDataTypeStr=Enum: OnOffNoReq  PortDimensions=1
  TelmThermoTankECOSet_TelmThermoTankECOSet : Inport  Port=315  OutDataTypeStr=Enum: NoReqOnOff  PortDimensions=1
  TelmThermoTankT_TelmThermoTankT : Inport  Port=316  OutDataTypeStr=EngT2  PortDimensions=1
  ThermHvacRecircReq_ThermHvacRecircReq : Inport  Port=317  OutMin=0  OutMax=100  OutDataTypeStr=Perc6  PortDimensions=1
  ThermHvacRecircReqEna_ThermHvacRecircReqEna : Inport  Port=318  OutDataTypeStr=Boolean  PortDimensions=1
  ThrdRowSeatHeatgActlPwrCnsLe_ThrdRowSeatHeatgActlPwrCnsLe : Inport  Port=319  OutDataTypeStr=UInt8  PortDimensions=1
  ThrdRowSeatHeatgActlPwrCnsRi_ThrdRowSeatHeatgActlPwrCnsRi : Inport  Port=320  OutDataTypeStr=UInt8  PortDimensions=1
  ThrdRowSeatHeatgDesPwrCnsLe_ThrdRowSeatHeatgDesPwrCnsLe : Inport  Port=321  OutDataTypeStr=UInt8  PortDimensions=1
  ThrdRowSeatHeatgDesPwrCnsRi_ThrdRowSeatHeatgDesPwrCnsRi : Inport  Port=322  OutDataTypeStr=UInt8  PortDimensions=1
  ThrdRowSeatVentnActlPwrCnsLe_ThrdRowSeatVentnActlPwrCnsLe : Inport  Port=323  OutDataTypeStr=UInt8  PortDimensions=1
  ThrdRowSeatVentnActlPwrCnsRi_ThrdRowSeatVentnActlPwrCnsRi : Inport  Port=324  OutDataTypeStr=UInt8  PortDimensions=1
  ThrdRowSeatVentnDesPwrCnsLe_ThrdRowSeatVentnDesPwrCnsLe : Inport  Port=325  OutDataTypeStr=UInt8  PortDimensions=1
  ThrdRowSeatVentnDesPwrCnsRi_ThrdRowSeatVentnDesPwrCnsRi : Inport  Port=326  OutDataTypeStr=UInt8  PortDimensions=1
  UseEvapReqTMinNew_UseEvapReqTMinNew : Inport  Port=327  OutDataTypeStr=Enum: OnOff1  PortDimensions=1
  VlvVoltFlt_VlvVoltFlt : Inport  Port=328  OutMin=0  OutMax=3  OutDataTypeStr=UInt2  PortDimensions=1
  WarnLvlMsg_WarnLvlMsg : Inport  Port=329  OutDataTypeStr=Enum: WarnLvl  PortDimensions=1
  WarnLvlMsg_WarnLvlMsg_IsUpdated : Inport  Port=330  OutDataTypeStr=boolean  PortDimensions=1
  WasRespLevel_WasRespLevel : Inport  Port=331  OutDataTypeStr=Enum: WasRespLevel  PortDimensions=1
  WasRespLevel_WasRespLevel_IsUpdated : Inport  Port=332  OutDataTypeStr=boolean  PortDimensions=1
  WaterDepthInformation_WaterDepthInformation : Inport  Port=333  OutDataTypeStr=Bus: WaterDepthInformation  PortDimensions=1  BusOutputAsStruct=on
  WeatherQualityAQI_WeatherQualityAQI : Inport  Port=334  OutMin=0  OutMax=1000  OutDataTypeStr=LineLenWithVehForBkp  PortDimensions=1
  AmbTOutOfVeh_AmbTOutOfVeh_merge : Merge
  AmbTRaw_Ngcc_AmbTRaw_merge : Merge
  CmptmtRelHum_Ngcc_CmptmtRelHum_merge : Merge
  CooltHeatrAvlStsForPrkgClima_CooltHeatrAvlStsForPrkgClima_merge : Merge
  EngT_Ngcc_EngT_merge : Merge
  FogOutOfVeh_FogOutOfVeh_merge : Merge
  HumOutOfVeh_HumOutOfVeh_merge : Merge
  HvBattCoolgPwrHeatgMgrThermPred_HvBattCoolgPwrHeatgMgrThermPred_merge : Merge
  IsgMotTInt_IsgMotTInt_merge : Merge
  MemWrForCooltHeatrActvnCtrl_MemWrForCooltHeatrActvnCtrl_merge : Merge
  MemWrForCooltHeatrCtrl_MemWrForCooltHeatrCtrl_merge : Merge
  MemWrForFuTInFuHeatrMdl_MemWrForFuTInFuHeatrMdl_merge : Merge
  MemWrForHeatgInpMgr_MemWrForHeatgInpMgr_merge : Merge
  MemWrForSnsrMdlCooltT_MemWrForSnsrMdlCooltT_merge : Merge
  SdbRxSigProc_Init : Subsystem
  SdbRxSigProc_Runnable_sys : Subsystem
  SunData_SunData_merge : Merge
  SunLoadOutOfVeh_SunLoadOutOfVeh_merge : Merge
  AmbTOutOfVeh_AmbTOutOfVeh : Outport  OutDataTypeStr=Bus: AmbTOutOfVeh  PortDimensions=1  BusOutputAsStruct=on
  AmbTRaw_Ngcc_AmbTRaw : Outport  Port=2  OutDataTypeStr=Bus: AmbTWithQly  PortDimensions=1  BusOutputAsStruct=on
  CmptmtRelHum_Ngcc_CmptmtRelHum : Outport  Port=3  OutDataTypeStr=Bus: CmptmRelHum  PortDimensions=1  BusOutputAsStruct=on
  CooltHeatrAvlStsForPrkgClima_CooltHeatrAvlStsForPrkgClima : Outport  Port=4  OutDataTypeStr=Bus: CooltHeatrAvlSts1  PortDimensions=1  BusOutputAsStruct=on
  EngT_Ngcc_EngT : Outport  Port=5  OutDataTypeStr=Bus: EngT  PortDimensions=1  BusOutputAsStruct=on
  FogOutOfVeh_FogOutOfVeh : Outport  Port=6  OutDataTypeStr=Bus: FogOutOfVeh  PortDimensions=1  BusOutputAsStruct=on
  HumOutOfVeh_HumOutOfVeh : Outport  Port=7  OutDataTypeStr=Bus: HumOutOfVeh  PortDimensions=1  BusOutputAsStruct=on
  HvBattCoolgPwrHeatgMgrThermPred_HvBattCoolgPwrHeatgMgrThermPred : Outport  Port=8  OutDataTypeStr=Bus: HvBattCoolgPwrHeatgMgrThermPred  PortDimensions=1  BusOutputAsStruct=on
  IsgMotTInt_IsgMotTInt : Outport  Port=9  OutDataTypeStr=T3  PortDimensions=1
  MemWrForCooltHeatrActvnCtrl_MemWrForCooltHeatrActvnCtrl : Outport  Port=10  OutDataTypeStr=Bus: MemRecForCooltHeatrActvnCtrl  PortDimensions=1  BusOutputAsStruct=on
  MemWrForCooltHeatrCtrl_MemWrForCooltHeatrCtrl : Outport  Port=11  OutDataTypeStr=Bus: MemRecForCooltHeatrCtrl  PortDimensions=1  BusOutputAsStruct=on